simulink model "Robot_Falling_Down_Stairs"  (R2024b)
settings: Solver=ode23t  StartTime=0.0  StopTime=4  RelTol=1e-3  AbsTol=1e-2  EnableMultiTasking=on
undefined symbols (not defined in the file): points, X, Y, Z
libraries: sm_lib x981, PC_GS_SSMB x30, nesl_utility x4
(part 4 of 22)

== system Robot_Falling_Down_Stairs/Sagte_Woef/FLLeg/Joint ==
  Revolute Joint1 : sm_lib/Joints/Revolute Joint  JointMode=Normal  CompositeWrenchDir=FollowerOnBase  CompositeWrenchFrame=BaseFrame  SenseConstraintForce=off  SenseConstraintTorque=off  SenseTotalForce=off  SenseTotalTorque=off  PositionTargetSpecify=on  PositionTargetPriority=High  PositionTargetValue=-50  PositionTargetValueUnits=deg  VelocityTargetSpecify=off  VelocityTargetPriority=High  VelocityTargetValue=0  VelocityTargetValueUnits=deg/s  EquilibriumPosition=0  EquilibriumPositionUnits=deg  SpringStiffness=0  SpringStiffnessUnits=N*m/deg  DampingCoefficient=1  DampingCoefficientUnits=N*m/(deg/s)  LowerLimitSpecify=off  LowerLimitBound=-90  LowerLimitBoundUnits=deg  LowerLimitStiffness=1e4  LowerLimitStiffnessUnits=N*m/deg  LowerLimitDamping=10  LowerLimitDampingUnits=N*m/(deg/s)  LowerLimitTransitionRegionWidth=0.1  LowerLimitTransitionRegionWidthUnits=deg  UpperLimitSpecify=off  UpperLimitBound=90  UpperLimitBoundUnits=deg  UpperLimitStiffness=1e4  UpperLimitStiffnessUnits=N*m/deg  UpperLimitDamping=10  UpperLimitDampingUnits=N*m/(deg/s)  UpperLimitTransitionRegionWidth=0.1  UpperLimitTransitionRegionWidthUnits=deg  TorqueActuationMode=NoTorque  MotionActuationMode=ComputedMotion  SensePosition=off  SenseVelocity=off  SenseAcceleration=off  SenseTorqueForce=off  SenseLowerLimitTorqueForce=off  SenseUpperLimitTorqueForce=off
  Rigid Transform2 : sm_lib/Frames and Transforms/Rigid Transform  RotationMethod=StandardAxis  FollAlignAxisA=+X  BaseAlignAxisA=+Y  FollAlignAxisB=+Y  BaseAlignAxisB=+Z  RotationStandardAxis=+X  RotationAngle=90  RotationAngleUnits=deg  RotationArbitraryAxis=[0 0 1]  RotationSequenceAxes=FollowerAxes  RotationSequence=XYX  RotationSequenceAngles=[0 0 0]  RotationSequenceAnglesUnits=deg  RotationMatrix=[1 0 0;0 1 0;0 0 1]  Quaternion=[1 0 0 0]  TranslationMethod=None  TranslationCartesianOffset=[0 0 0]  TranslationCartesianOffsetUnits=m  TranslationStandardAxis=+Z  TranslationStandardOffset=0  TranslationStandardOffsetUnits=m  TranslationROffset=0  TranslationROffsetUnits=m  TranslationThetaOffset=0  TranslationThetaOffsetUnits=deg  TranslationZOffset=0  TranslationZOffsetUnits=m
  Rigid Transform3 : sm_lib/Frames and Transforms/Rigid Transform  RotationMethod=StandardAxis  FollAlignAxisA=+X  BaseAlignAxisA=+Y  FollAlignAxisB=+Y  BaseAlignAxisB=+Z  RotationStandardAxis=+X  RotationAngle=90  RotationAngleUnits=deg  RotationArbitraryAxis=[0 0 1]  RotationSequenceAxes=FollowerAxes  RotationSequence=XYX  RotationSequenceAngles=[0 0 0]  RotationSequenceAnglesUnits=deg  RotationMatrix=[1 0 0;0 1 0;0 0 1]  Quaternion=[1 0 0 0]  TranslationMethod=None  TranslationCartesianOffset=[0 0 0]  TranslationCartesianOffsetUnits=m  TranslationStandardAxis=+Z  TranslationStandardOffset=0  TranslationStandardOffsetUnits=m  TranslationROffset=0  TranslationROffsetUnits=m  TranslationThetaOffset=0  TranslationThetaOffsetUnits=deg  TranslationZOffset=0  TranslationZOffsetUnits=m
  Conn1 : PMIOPort  Side=Right
  Top : PMIOPort  Port=2  Side=Left
  connections:
  Rigid Transform3/RConn1 -- Top/RConn1
  Rigid Transform2/LConn1 -- Revolute Joint1/LConn1
  Revolute Joint1/RConn1 -- Rigid Transform3/LConn1
  Conn1/RConn1 -- Rigid Transform2/RConn1

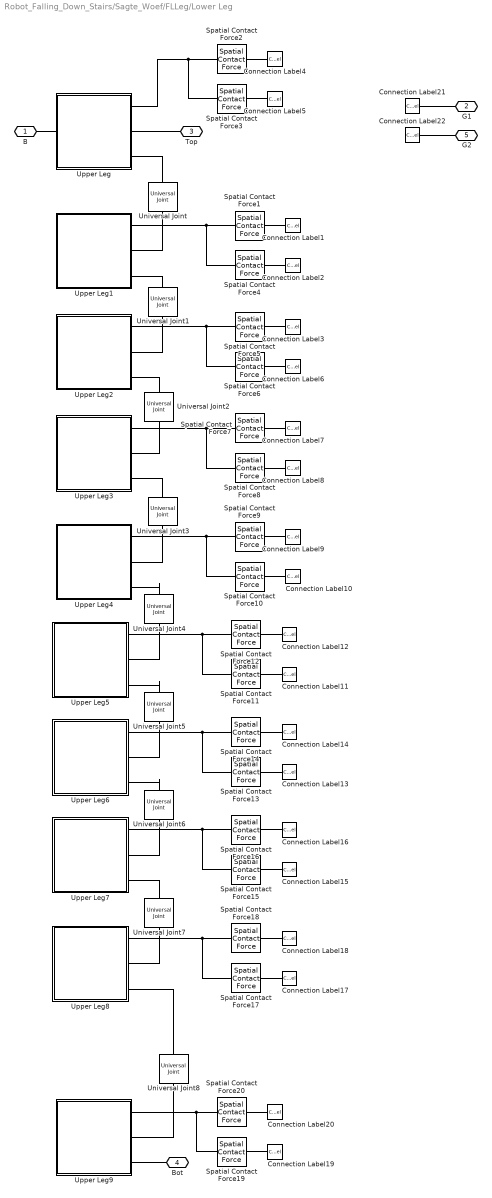
== system Robot_Falling_Down_Stairs/Sagte_Woef/FLLeg/Lower Leg ==
  Connection Label{1,3..4,7,9,12,14,16,18,20..21} (11 alike) : ConnectionLabel  Label=G1
  Connection Label{2,5..6,8,10..11,13,15,17,19,22} (11 alike) : ConnectionLabel  Label=G2
  Spatial Contact Force{1..20} (20 alike) : sm_lib/Forces and Torques/Spatial Contact Force  NormalForceType=SmoothSpringDamper  NormalStiffness=1e6  NormalStiffnessUnits=N/m  NormalDamping=1e3  NormalDampingUnits=N/(m/s)  NormalTransitionRegionWidth=1e-2  NormalTransitionRegionWidthUnits=m  FrictionType=SmoothStickSlip  CoefficientOfStaticFriction=0.5  CoefficientOfDynamicFriction=0.3  FrictionalCriticalVelocity=1e-3  FrictionalCriticalVelocityUnits=m/s  SenseContactSignal=off  SensePenetrationDepth=off  SenseSeparationDistance=off  SenseNormalForceMagnitude=off  SenseFrictionalForceMagnitude=off  SenseNormalVelocity=off  SenseTangentialVelocity=off  SenseContactBaseRotation=off  SenseContactBaseTranslation=off  SenseContactFollowerRotation=off  SenseContactFollowerTranslation=off  ZeroCrossDetectContactStartAndEnd=off
  Universal Joint{,1..8} (9 alike) : sm_lib/Joints/Universal Joint  JointMode=Normal  CompositeWrenchDir=FollowerOnBase  CompositeWrenchFrame=BaseFrame  SenseConstraintForce=off  SenseConstraintTorque=off  SenseTotalForce=off  SenseTotalTorque=off  RxPositionTargetSpecify=off  RxPositionTargetPriority=High  RxPositionTargetValue=0  RxPositionTargetValueUnits=deg  RxVelocityTargetSpecify=off  RxVelocityTargetPriority=High  RxVelocityTargetValue=0  RxVelocityTargetValueUnits=deg/s  RxEquilibriumPosition=0  RxEquilibriumPositionUnits=deg  RxSpringStiffness=100  RxSpringStiffnessUnits=N*m/deg  RxDampingCoefficient=.1  RxDampingCoefficientUnits=N*m/(deg/s)  RxLowerLimitSpecify=off  RxLowerLimitBound=-90  RxLowerLimitBoundUnits=deg  RxLowerLimitStiffness=1e4  RxLowerLimitStiffnessUnits=N*m/deg  RxLowerLimitDamping=10  RxLowerLimitDampingUnits=N*m/(deg/s)  RxLowerLimitTransitionRegionWidth=0.1  RxLowerLimitTransitionRegionWidthUnits=deg  RxUpperLimitSpecify=off  RxUpperLimitBound=90  RxUpperLimitBoundUnits=deg  RxUpperLimitStiffness=1e4  RxUpperLimitStiffnessUnits=N*m/deg  RxUpperLimitDamping=10  RxUpperLimitDampingUnits=N*m/(deg/s)  RxUpperLimitTransitionRegionWidth=0.1  RxUpperLimitTransitionRegionWidthUnits=deg  RxTorqueActuationMode=NoTorque  RxMotionActuationMode=ComputedMotion  RxSensePosition=off  RxSenseVelocity=off  RxSenseAcceleration=off  RxSenseTorqueForce=off  RxSenseLowerLimitTorqueForce=off  RxSenseUpperLimitTorqueForce=off  RyPositionTargetSpecify=off  RyPositionTargetPriority=High  RyPositionTargetValue=0  RyPositionTargetValueUnits=deg  RyVelocityTargetSpecify=off  RyVelocityTargetPriority=High  RyVelocityTargetValue=0  RyVelocityTargetValueUnits=deg/s  RyEquilibriumPosition=0  RyEquilibriumPositionUnits=deg  RySpringStiffness=100  RySpringStiffnessUnits=N*m/deg  RyDampingCoefficient=.1  RyDampingCoefficientUnits=N*m/(deg/s)  RyLowerLimitSpecify=off  RyLowerLimitBound=-90  RyLowerLimitBoundUnits=deg  RyLowerLimitStiffness=1e4  RyLowerLimitStiffnessUnits=N*m/deg  RyLowerLimitDamping=10  RyLowerLimitDampingUnits=N*m/(deg/s)  RyLowerLimitTransitionRegionWidth=0.1  RyLowerLimitTransitionRegionWidthUnits=deg  RyUpperLimitSpecify=off  RyUpperLimitBound=90  RyUpperLimitBoundUnits=deg  RyUpperLimitStiffness=1e4  RyUpperLimitStiffnessUnits=N*m/deg  RyUpperLimitDamping=10  RyUpperLimitDampingUnits=N*m/(deg/s)  RyUpperLimitTransitionRegionWidth=0.1  RyUpperLimitTransitionRegionWidthUnits=deg  RyTorqueActuationMode=NoTorque  RyMotionActuationMode=ComputedMotion  RySensePosition=off  RySenseVelocity=off  RySenseAcceleration=off  RySenseTorqueForce=off  RySenseLowerLimitTorqueForce=off  RySenseUpperLimitTorqueForce=off
  Upper Leg : Subsystem  AncestorBlock=PC_GS_SSMB/PC_Cylinder_F  mask{radius=30, height=40, numPoints=9*3*3, numCircumferencePoints=0, numFacePoints=20, patchColor=[0.1940, 0.4840, 0.5560], markerColor=[0 0.4470 0.7410]}
      mask prompts: radius "Radius of the cylider [mm]"; height "Height of the cylinder [mm]"; numPoints "Total number of points for the curved surface using the Fibonacci lattice"; numCircumferencePoints "Number of points on the circumference of each disc"; numFacePoints "Number of points on each disc surface using the Fibonacci lattice"; patchColor "RGB color for the patches"; markerColor "RGB color for the markers"
      mask init:
      | [points, imageMatrix] = generateCylinderPointCloudFibonacci(radius, height, numPoints, numCircumferencePoints, numFacePoints, patchColor, markerColor);
      mask Display: image(imageMatrix);
  Upper Leg1 : Subsystem  AncestorBlock=PC_GS_SSMB/PC_Cylinder_F  mask{radius=30, height=40, numPoints=9*3*3, numCircumferencePoints=0, numFacePoints=0, patchColor=[0.1940, 0.4840, 0.5560], markerColor=[0 0.4470 0.7410]}
      mask prompts: radius "Radius of the cylider [mm]"; height "Height of the cylinder [mm]"; numPoints "Total number of points for the curved surface using the Fibonacci lattice"; numCircumferencePoints "Number of points on the circumference of each disc"; numFacePoints "Number of points on each disc surface using the Fibonacci lattice"; patchColor "RGB color for the patches"; markerColor "RGB color for the markers"
      mask init:
      | [points, imageMatrix] = generateCylinderPointCloudFibonacci(radius, height, numPoints, numCircumferencePoints, numFacePoints, patchColor, markerColor);
      mask Display: image(imageMatrix);
  Upper Leg2 : Subsystem  AncestorBlock=PC_GS_SSMB/PC_Cylinder_F  mask{radius=30, height=40, numPoints=9*3*3, numCircumferencePoints=0, numFacePoints=0, patchColor=[0.1940, 0.4840, 0.5560], markerColor=[0 0.4470 0.7410]}
      mask prompts: radius "Radius of the cylider [mm]"; height "Height of the cylinder [mm]"; numPoints "Total number of points for the curved surface using the Fibonacci lattice"; numCircumferencePoints "Number of points on the circumference of each disc"; numFacePoints "Number of points on each disc surface using the Fibonacci lattice"; patchColor "RGB color for the patches"; markerColor "RGB color for the markers"
      mask init:
      | [points, imageMatrix] = generateCylinderPointCloudFibonacci(radius, height, numPoints, numCircumferencePoints, numFacePoints, patchColor, markerColor);
      mask Display: image(imageMatrix);
  Upper Leg3 : Subsystem  AncestorBlock=PC_GS_SSMB/PC_Cylinder_F  mask{radius=30, height=40, numPoints=9*3*3, numCircumferencePoints=0, numFacePoints=0, patchColor=[0.1940, 0.4840, 0.5560], markerColor=[0 0.4470 0.7410]}
      mask prompts: radius "Radius of the cylider [mm]"; height "Height of the cylinder [mm]"; numPoints "Total number of points for the curved surface using the Fibonacci lattice"; numCircumferencePoints "Number of points on the circumference of each disc"; numFacePoints "Number of points on each disc surface using the Fibonacci lattice"; patchColor "RGB color for the patches"; markerColor "RGB color for the markers"
      mask init:
      | [points, imageMatrix] = generateCylinderPointCloudFibonacci(radius, height, numPoints, numCircumferencePoints, numFacePoints, patchColor, markerColor);
      mask Display: image(imageMatrix);
  Upper Leg4 : Subsystem  AncestorBlock=PC_GS_SSMB/PC_Cylinder_F  mask{radius=30, height=40, numPoints=9*3*3, numCircumferencePoints=0, numFacePoints=0, patchColor=[0.1940, 0.4840, 0.5560], markerColor=[0 0.4470 0.7410]}
      mask prompts: radius "Radius of the cylider [mm]"; height "Height of the cylinder [mm]"; numPoints "Total number of points for the curved surface using the Fibonacci lattice"; numCircumferencePoints "Number of points on the circumference of each disc"; numFacePoints "Number of points on each disc surface using the Fibonacci lattice"; patchColor "RGB color for the patches"; markerColor "RGB color for the markers"
      mask init:
      | [points, imageMatrix] = generateCylinderPointCloudFibonacci(radius, height, numPoints, numCircumferencePoints, numFacePoints, patchColor, markerColor);
      mask Display: image(imageMatrix);
  Upper Leg5 : Subsystem  AncestorBlock=PC_GS_SSMB/PC_Cylinder_F  mask{radius=30, height=40, numPoints=9*3*3, numCircumferencePoints=0, numFacePoints=0, patchColor=[0.1940, 0.4840, 0.5560], markerColor=[0 0.4470 0.7410]}
      mask prompts: radius "Radius of the cylider [mm]"; height "Height of the cylinder [mm]"; numPoints "Total number of points for the curved surface using the Fibonacci lattice"; numCircumferencePoints "Number of points on the circumference of each disc"; numFacePoints "Number of points on each disc surface using the Fibonacci lattice"; patchColor "RGB color for the patches"; markerColor "RGB color for the markers"
      mask init:
      | [points, imageMatrix] = generateCylinderPointCloudFibonacci(radius, height, numPoints, numCircumferencePoints, numFacePoints, patchColor, markerColor);
      mask Display: image(imageMatrix);
  Upper Leg6 : Subsystem  AncestorBlock=PC_GS_SSMB/PC_Cylinder_F  mask{radius=30, height=40, numPoints=9*3*3, numCircumferencePoints=0, numFacePoints=0, patchColor=[0.1940, 0.4840, 0.5560], markerColor=[0 0.4470 0.7410]}
      mask prompts: radius "Radius of the cylider [mm]"; height "Height of the cylinder [mm]"; numPoints "Total number of points for the curved surface using the Fibonacci lattice"; numCircumferencePoints "Number of points on the circumference of each disc"; numFacePoints "Number of points on each disc surface using the Fibonacci lattice"; patchColor "RGB color for the patches"; markerColor "RGB color for the markers"
      mask init:
      | [points, imageMatrix] = generateCylinderPointCloudFibonacci(radius, height, numPoints, numCircumferencePoints, numFacePoints, patchColor, markerColor);
      mask Display: image(imageMatrix);
  Upper Leg7 : Subsystem  AncestorBlock=PC_GS_SSMB/PC_Cylinder_F  mask{radius=30, height=40, numPoints=9*3*3, numCircumferencePoints=0, numFacePoints=0, patchColor=[0.1940, 0.4840, 0.5560], markerColor=[0 0.4470 0.7410]}
      mask prompts: radius "Radius of the cylider [mm]"; height "Height of the cylinder [mm]"; numPoints "Total number of points for the curved surface using the Fibonacci lattice"; numCircumferencePoints "Number of points on the circumference of each disc"; numFacePoints "Number of points on each disc surface using the Fibonacci lattice"; patchColor "RGB color for the patches"; markerColor "RGB color for the markers"
      mask init:
      | [points, imageMatrix] = generateCylinderPointCloudFibonacci(radius, height, numPoints, numCircumferencePoints, numFacePoints, patchColor, markerColor);
      mask Display: image(imageMatrix);
  Upper Leg8 : Subsystem  AncestorBlock=PC_GS_SSMB/PC_Cylinder_F  mask{radius=30, height=40, numPoints=9*3*3, numCircumferencePoints=0, numFacePoints=0, patchColor=[0.1940, 0.4840, 0.5560], markerColor=[0 0.4470 0.7410]}
      mask prompts: radius "Radius of the cylider [mm]"; height "Height of the cylinder [mm]"; numPoints "Total number of points for the curved surface using the Fibonacci lattice"; numCircumferencePoints "Number of points on the circumference of each disc"; numFacePoints "Number of points on each disc surface using the Fibonacci lattice"; patchColor "RGB color for the patches"; markerColor "RGB color for the markers"
      mask init:
      | [points, imageMatrix] = generateCylinderPointCloudFibonacci(radius, height, numPoints, numCircumferencePoints, numFacePoints, patchColor, markerColor);
      mask Display: image(imageMatrix);
  Upper Leg9 : Subsystem  AncestorBlock=PC_GS_SSMB/PC_Cylinder_F  mask{radius=30, height=40, numPoints=9*3*3, numCircumferencePoints=0, numFacePoints=20, patchColor=[0.1940, 0.4840, 0.5560], markerColor=[0 0.4470 0.7410]}
      mask prompts: radius "Radius of the cylider [mm]"; height "Height of the cylinder [mm]"; numPoints "Total number of points for the curved surface using the Fibonacci lattice"; numCircumferencePoints "Number of points on the circumference of each disc"; numFacePoints "Number of points on each disc surface using the Fibonacci lattice"; patchColor "RGB color for the patches"; markerColor "RGB color for the markers"
      mask init:
      | [points, imageMatrix] = generateCylinderPointCloudFibonacci(radius, height, numPoints, numCircumferencePoints, numFacePoints, patchColor, markerColor);
      mask Display: image(imageMatrix);
  B : PMIOPort  Side=Left
  G1 : PMIOPort  Port=2  Side=Right
  Top : PMIOPort  Port=3  Side=Right
  Bot : PMIOPort  Port=4  Side=Right
  G2 : PMIOPort  Port=5  Side=Right
  connections:
  Connection Label22/LConn1 -- G2/RConn1
  Connection Label21/LConn1 -- G1/RConn1
  Universal Joint1/LConn1 -- Upper Leg1/RConn3
  Universal Joint2/LConn1 -- Upper Leg2/RConn3
  Universal Joint3/LConn1 -- Upper Leg3/RConn3
  Universal Joint4/LConn1 -- Upper Leg4/RConn3
  Universal Joint5/LConn1 -- Upper Leg5/RConn3
  Universal Joint6/LConn1 -- Upper Leg6/RConn3
  Universal Joint7/LConn1 -- Upper Leg7/RConn3
  Universal Joint8/LConn1 -- Upper Leg8/RConn3
  Spatial Contact Force19/LConn1 -- Spatial Contact Force20/LConn1 -- Upper Leg9/RConn1
  Connection Label19/LConn1 -- Spatial Contact Force19/RConn1
  Spatial Contact Force20/RConn1 -- Connection Label20/LConn1
  Upper Leg9/RConn2 -- Universal Joint8/RConn1
  Spatial Contact Force18/RConn1 -- Connection Label18/LConn1
  Connection Label17/LConn1 -- Spatial Contact Force17/RConn1
  Universal Joint7/RConn1 -- Upper Leg8/RConn2
  Spatial Contact Force17/LConn1 -- Upper Leg8/RConn1 -- Spatial Contact Force18/LConn1
  Universal Joint6/RConn1 -- Upper Leg7/RConn2
  Spatial Contact Force16/RConn1 -- Connection Label16/LConn1
  Connection Label15/LConn1 -- Spatial Contact Force15/RConn1
  Upper Leg7/RConn1 -- Spatial Contact Force15/LConn1 -- Spatial Contact Force16/LConn1
  Connection Label13/LConn1 -- Spatial Contact Force13/RConn1
  Spatial Contact Force14/RConn1 -- Connection Label14/LConn1
  Universal Joint5/RConn1 -- Upper Leg6/RConn2
  Spatial Contact Force13/LConn1 -- Upper Leg6/RConn1 -- Spatial Contact Force14/LConn1
  Universal Joint4/RConn1 -- Upper Leg5/RConn2
  Connection Label11/LConn1 -- Spatial Contact Force11/RConn1
  Spatial Contact Force12/RConn1 -- Connection Label12/LConn1
  Upper Leg5/RConn1 -- Spatial Contact Force11/LConn1 -- Spatial Contact Force12/LConn1
  Spatial Contact Force9/RConn1 -- Connection Label9/LConn1
  Universal Joint3/RConn1 -- Upper Leg4/RConn2
  Connection Label10/LConn1 -- Spatial Contact Force10/RConn1
  Spatial Contact Force10/LConn1 -- Upper Leg4/RConn1 -- Spatial Contact Force9/LConn1
  Universal Joint2/RConn1 -- Upper Leg3/RConn2
  Connection Label8/LConn1 -- Spatial Contact Force8/RConn1
  Spatial Contact Force7/RConn1 -- Connection Label7/LConn1
  Upper Leg3/RConn1 -- Spatial Contact Force8/LConn1 -- Spatial Contact Force7/LConn1
  Universal Joint1/RConn1 -- Upper Leg2/RConn2
  Spatial Contact Force5/RConn1 -- Connection Label3/LConn1
  Connection Label6/LConn1 -- Spatial Contact Force6/RConn1
  Spatial Contact Force6/LConn1 -- Upper Leg2/RConn1 -- Spatial Contact Force5/LConn1
  Connection Label2/LConn1 -- Spatial Contact Force4/RConn1
  Spatial Contact Force1/RConn1 -- Connection Label1/LConn1
  Upper Leg1/RConn1 -- Spatial Contact Force4/LConn1 -- Spatial Contact Force1/LConn1
  Universal Joint/RConn1 -- Upper Leg1/RConn2
  Upper Leg/RConn3 -- Universal Joint/LConn1
  Connection Label5/LConn1 -- Spatial Contact Force3/RConn1
  Spatial Contact Force2/RConn1 -- Connection Label4/LConn1
  Upper Leg/RConn1 -- Spatial Contact Force2/LConn1 -- Spatial Contact Force3/LConn1
  Bot/RConn1 -- Upper Leg9/RConn3
  Upper Leg/LConn1 -- B/RConn1
  Upper Leg/RConn2 -- Top/RConn1

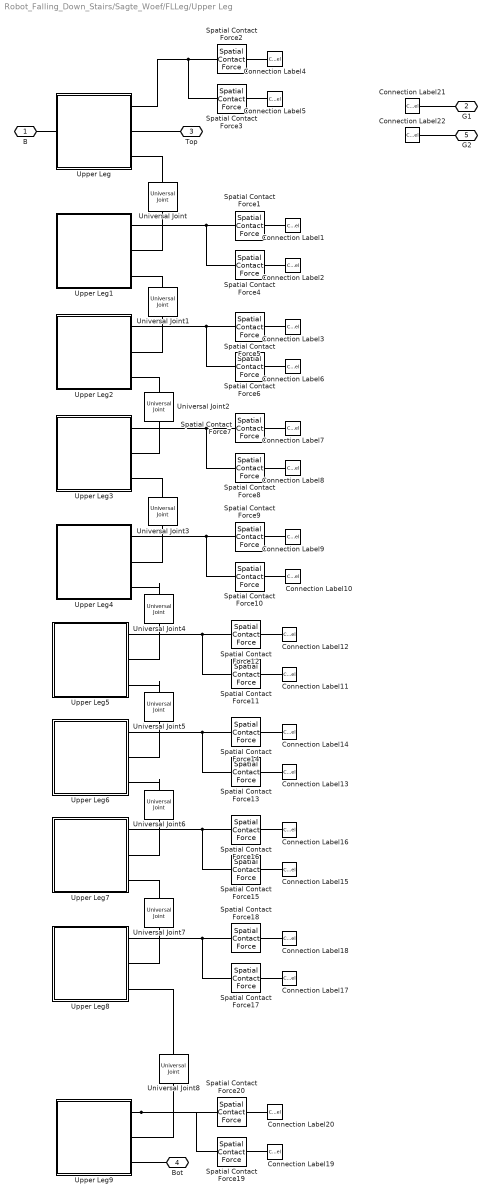
== system Robot_Falling_Down_Stairs/Sagte_Woef/FLLeg/Upper Leg ==
  Connection Label{1,3..4,7,9,12,14,16,18,20..21} (11 alike) : ConnectionLabel  Label=G1
  Connection Label{2,5..6,8,10..11,13,15,17,19,22} (11 alike) : ConnectionLabel  Label=G2
  Spatial Contact Force{1..20} (20 alike) : sm_lib/Forces and Torques/Spatial Contact Force  NormalForceType=SmoothSpringDamper  NormalStiffness=1e6  NormalStiffnessUnits=N/m  NormalDamping=1e3  NormalDampingUnits=N/(m/s)  NormalTransitionRegionWidth=1e-2  NormalTransitionRegionWidthUnits=m  FrictionType=SmoothStickSlip  CoefficientOfStaticFriction=0.5  CoefficientOfDynamicFriction=0.3  FrictionalCriticalVelocity=1e-3  FrictionalCriticalVelocityUnits=m/s  SenseContactSignal=off  SensePenetrationDepth=off  SenseSeparationDistance=off  SenseNormalForceMagnitude=off  SenseFrictionalForceMagnitude=off  SenseNormalVelocity=off  SenseTangentialVelocity=off  SenseContactBaseRotation=off  SenseContactBaseTranslation=off  SenseContactFollowerRotation=off  SenseContactFollowerTranslation=off  ZeroCrossDetectContactStartAndEnd=off
  Universal Joint{,1..8} (9 alike) : sm_lib/Joints/Universal Joint  JointMode=Normal  CompositeWrenchDir=FollowerOnBase  CompositeWrenchFrame=BaseFrame  SenseConstraintForce=off  SenseConstraintTorque=off  SenseTotalForce=off  SenseTotalTorque=off  RxPositionTargetSpecify=off  RxPositionTargetPriority=High  RxPositionTargetValue=0  RxPositionTargetValueUnits=deg  RxVelocityTargetSpecify=off  RxVelocityTargetPriority=High  RxVelocityTargetValue=0  RxVelocityTargetValueUnits=deg/s  RxEquilibriumPosition=0  RxEquilibriumPositionUnits=deg  RxSpringStiffness=100  RxSpringStiffnessUnits=N*m/deg  RxDampingCoefficient=.1  RxDampingCoefficientUnits=N*m/(deg/s)  RxLowerLimitSpecify=off  RxLowerLimitBound=-90  RxLowerLimitBoundUnits=deg  RxLowerLimitStiffness=1e4  RxLowerLimitStiffnessUnits=N*m/deg  RxLowerLimitDamping=10  RxLowerLimitDampingUnits=N*m/(deg/s)  RxLowerLimitTransitionRegionWidth=0.1  RxLowerLimitTransitionRegionWidthUnits=deg  RxUpperLimitSpecify=off  RxUpperLimitBound=90  RxUpperLimitBoundUnits=deg  RxUpperLimitStiffness=1e4  RxUpperLimitStiffnessUnits=N*m/deg  RxUpperLimitDamping=10  RxUpperLimitDampingUnits=N*m/(deg/s)  RxUpperLimitTransitionRegionWidth=0.1  RxUpperLimitTransitionRegionWidthUnits=deg  RxTorqueActuationMode=NoTorque  RxMotionActuationMode=ComputedMotion  RxSensePosition=off  RxSenseVelocity=off  RxSenseAcceleration=off  RxSenseTorqueForce=off  RxSenseLowerLimitTorqueForce=off  RxSenseUpperLimitTorqueForce=off  RyPositionTargetSpecify=off  RyPositionTargetPriority=High  RyPositionTargetValue=0  RyPositionTargetValueUnits=deg  RyVelocityTargetSpecify=off  RyVelocityTargetPriority=High  RyVelocityTargetValue=0  RyVelocityTargetValueUnits=deg/s  RyEquilibriumPosition=0  RyEquilibriumPositionUnits=deg  RySpringStiffness=100  RySpringStiffnessUnits=N*m/deg  RyDampingCoefficient=.1  RyDampingCoefficientUnits=N*m/(deg/s)  RyLowerLimitSpecify=off  RyLowerLimitBound=-90  RyLowerLimitBoundUnits=deg  RyLowerLimitStiffness=1e4  RyLowerLimitStiffnessUnits=N*m/deg  RyLowerLimitDamping=10  RyLowerLimitDampingUnits=N*m/(deg/s)  RyLowerLimitTransitionRegionWidth=0.1  RyLowerLimitTransitionRegionWidthUnits=deg  RyUpperLimitSpecify=off  RyUpperLimitBound=90  RyUpperLimitBoundUnits=deg  RyUpperLimitStiffness=1e4  RyUpperLimitStiffnessUnits=N*m/deg  RyUpperLimitDamping=10  RyUpperLimitDampingUnits=N*m/(deg/s)  RyUpperLimitTransitionRegionWidth=0.1  RyUpperLimitTransitionRegionWidthUnits=deg  RyTorqueActuationMode=NoTorque  RyMotionActuationMode=ComputedMotion  RySensePosition=off  RySenseVelocity=off  RySenseAcceleration=off  RySenseTorqueForce=off  RySenseLowerLimitTorqueForce=off  RySenseUpperLimitTorqueForce=off
  Upper Leg : Subsystem  AncestorBlock=PC_GS_SSMB/PC_Cylinder_F  mask{radius=40, height=30, numPoints=9*3*3, numCircumferencePoints=0, numFacePoints=20, patchColor=[0.4940, 0.1840, 0.5560], markerColor=[0 0.4470 0.7410]}
      mask prompts: radius "Radius of the cylider [mm]"; height "Height of the cylinder [mm]"; numPoints "Total number of points for the curved surface using the Fibonacci lattice"; numCircumferencePoints "Number of points on the circumference of each disc"; numFacePoints "Number of points on each disc surface using the Fibonacci lattice"; patchColor "RGB color for the patches"; markerColor "RGB color for the markers"
      mask init:
      | [points, imageMatrix] = generateCylinderPointCloudFibonacci(radius, height, numPoints, numCircumferencePoints, numFacePoints, patchColor, markerColor);
      mask Display: image(imageMatrix);
  Upper Leg1 : Subsystem  AncestorBlock=PC_GS_SSMB/PC_Cylinder_F  mask{radius=40, height=30, numPoints=9*3*3, numCircumferencePoints=0, numFacePoints=0, patchColor=[0.4940, 0.1840, 0.5560], markerColor=[0 0.4470 0.7410]}
      mask prompts: radius "Radius of the cylider [mm]"; height "Height of the cylinder [mm]"; numPoints "Total number of points for the curved surface using the Fibonacci lattice"; numCircumferencePoints "Number of points on the circumference of each disc"; numFacePoints "Number of points on each disc surface using the Fibonacci lattice"; patchColor "RGB color for the patches"; markerColor "RGB color for the markers"
      mask init:
      | [points, imageMatrix] = generateCylinderPointCloudFibonacci(radius, height, numPoints, numCircumferencePoints, numFacePoints, patchColor, markerColor);
      mask Display: image(imageMatrix);
  Upper Leg2 : Subsystem  AncestorBlock=PC_GS_SSMB/PC_Cylinder_F  mask{radius=40, height=30, numPoints=9*3*3, numCircumferencePoints=0, numFacePoints=0, patchColor=[0.4940, 0.1840, 0.5560], markerColor=[0 0.4470 0.7410]}
      mask prompts: radius "Radius of the cylider [mm]"; height "Height of the cylinder [mm]"; numPoints "Total number of points for the curved surface using the Fibonacci lattice"; numCircumferencePoints "Number of points on the circumference of each disc"; numFacePoints "Number of points on each disc surface using the Fibonacci lattice"; patchColor "RGB color for the patches"; markerColor "RGB color for the markers"
      mask init:
      | [points, imageMatrix] = generateCylinderPointCloudFibonacci(radius, height, numPoints, numCircumferencePoints, numFacePoints, patchColor, markerColor);
      mask Display: image(imageMatrix);
  Upper Leg3 : Subsystem  AncestorBlock=PC_GS_SSMB/PC_Cylinder_F  mask{radius=40, height=30, numPoints=9*3*3, numCircumferencePoints=0, numFacePoints=0, patchColor=[0.4940, 0.1840, 0.5560], markerColor=[0 0.4470 0.7410]}
      mask prompts: radius "Radius of the cylider [mm]"; height "Height of the cylinder [mm]"; numPoints "Total number of points for the curved surface using the Fibonacci lattice"; numCircumferencePoints "Number of points on the circumference of each disc"; numFacePoints "Number of points on each disc surface using the Fibonacci lattice"; patchColor "RGB color for the patches"; markerColor "RGB color for the markers"
      mask init:
      | [points, imageMatrix] = generateCylinderPointCloudFibonacci(radius, height, numPoints, numCircumferencePoints, numFacePoints, patchColor, markerColor);
      mask Display: image(imageMatrix);
  Upper Leg4 : Subsystem  AncestorBlock=PC_GS_SSMB/PC_Cylinder_F  mask{radius=40, height=30, numPoints=9*3*3, numCircumferencePoints=0, numFacePoints=0, patchColor=[0.4940, 0.1840, 0.5560], markerColor=[0 0.4470 0.7410]}
      mask prompts: radius "Radius of the cylider [mm]"; height "Height of the cylinder [mm]"; numPoints "Total number of points for the curved surface using the Fibonacci lattice"; numCircumferencePoints "Number of points on the circumference of each disc"; numFacePoints "Number of points on each disc surface using the Fibonacci lattice"; patchColor "RGB color for the patches"; markerColor "RGB color for the markers"
      mask init:
      | [points, imageMatrix] = generateCylinderPointCloudFibonacci(radius, height, numPoints, numCircumferencePoints, numFacePoints, patchColor, markerColor);
      mask Display: image(imageMatrix);
  Upper Leg5 : Subsystem  AncestorBlock=PC_GS_SSMB/PC_Cylinder_F  mask{radius=40, height=30, numPoints=9*3*3, numCircumferencePoints=0, numFacePoints=0, patchColor=[0.4940, 0.1840, 0.5560], markerColor=[0 0.4470 0.7410]}
      mask prompts: radius "Radius of the cylider [mm]"; height "Height of the cylinder [mm]"; numPoints "Total number of points for the curved surface using the Fibonacci lattice"; numCircumferencePoints "Number of points on the circumference of each disc"; numFacePoints "Number of points on each disc surface using the Fibonacci lattice"; patchColor "RGB color for the patches"; markerColor "RGB color for the markers"
      mask init:
      | [points, imageMatrix] = generateCylinderPointCloudFibonacci(radius, height, numPoints, numCircumferencePoints, numFacePoints, patchColor, markerColor);
      mask Display: image(imageMatrix);
  Upper Leg6 : Subsystem  AncestorBlock=PC_GS_SSMB/PC_Cylinder_F  mask{radius=40, height=30, numPoints=9*3*3, numCircumferencePoints=0, numFacePoints=0, patchColor=[0.4940, 0.1840, 0.5560], markerColor=[0 0.4470 0.7410]}
      mask prompts: radius "Radius of the cylider [mm]"; height "Height of the cylinder [mm]"; numPoints "Total number of points for the curved surface using the Fibonacci lattice"; numCircumferencePoints "Number of points on the circumference of each disc"; numFacePoints "Number of points on each disc surface using the Fibonacci lattice"; patchColor "RGB color for the patches"; markerColor "RGB color for the markers"
      mask init:
      | [points, imageMatrix] = generateCylinderPointCloudFibonacci(radius, height, numPoints, numCircumferencePoints, numFacePoints, patchColor, markerColor);
      mask Display: image(imageMatrix);
  Upper Leg7 : Subsystem  AncestorBlock=PC_GS_SSMB/PC_Cylinder_F  mask{radius=40, height=30, numPoints=9*3*3, numCircumferencePoints=0, numFacePoints=0, patchColor=[0.4940, 0.1840, 0.5560], markerColor=[0 0.4470 0.7410]}
      mask prompts: radius "Radius of the cylider [mm]"; height "Height of the cylinder [mm]"; numPoints "Total number of points for the curved surface using the Fibonacci lattice"; numCircumferencePoints "Number of points on the circumference of each disc"; numFacePoints "Number of points on each disc surface using the Fibonacci lattice"; patchColor "RGB color for the patches"; markerColor "RGB color for the markers"
      mask init:
      | [points, imageMatrix] = generateCylinderPointCloudFibonacci(radius, height, numPoints, numCircumferencePoints, numFacePoints, patchColor, markerColor);
      mask Display: image(imageMatrix);
  Upper Leg8 : Subsystem  AncestorBlock=PC_GS_SSMB/PC_Cylinder_F  mask{radius=40, height=30, numPoints=9*3*3, numCircumferencePoints=0, numFacePoints=0, patchColor=[0.4940, 0.1840, 0.5560], markerColor=[0 0.4470 0.7410]}
      mask prompts: radius "Radius of the cylider [mm]"; height "Height of the cylinder [mm]"; numPoints "Total number of points for the curved surface using the Fibonacci lattice"; numCircumferencePoints "Number of points on the circumference of each disc"; numFacePoints "Number of points on each disc surface using the Fibonacci lattice"; patchColor "RGB color for the patches"; markerColor "RGB color for the markers"
      mask init:
      | [points, imageMatrix] = generateCylinderPointCloudFibonacci(radius, height, numPoints, numCircumferencePoints, numFacePoints, patchColor, markerColor);
      mask Display: image(imageMatrix);
  Upper Leg9 : Subsystem  AncestorBlock=PC_GS_SSMB/PC_Cylinder_F  mask{radius=40, height=30, numPoints=9*3*3, numCircumferencePoints=0, numFacePoints=20, patchColor=[0.4940, 0.1840, 0.5560], markerColor=[0 0.4470 0.7410]}
      mask prompts: radius "Radius of the cylider [mm]"; height "Height of the cylinder [mm]"; numPoints "Total number of points for the curved surface using the Fibonacci lattice"; numCircumferencePoints "Number of points on the circumference of each disc"; numFacePoints "Number of points on each disc surface using the Fibonacci lattice"; patchColor "RGB color for the patches"; markerColor "RGB color for the markers"
      mask init:
      | [points, imageMatrix] = generateCylinderPointCloudFibonacci(radius, height, numPoints, numCircumferencePoints, numFacePoints, patchColor, markerColor);
      mask Display: image(imageMatrix);
  B : PMIOPort  Side=Left
  G1 : PMIOPort  Port=2  Side=Right
  Top : PMIOPort  Port=3  Side=Right
  Bot : PMIOPort  Port=4  Side=Right
  G2 : PMIOPort  Port=5  Side=Right
  connections:
  Upper Leg/RConn2 -- Top/RConn1
  Upper Leg/LConn1 -- B/RConn1
  Bot/RConn1 -- Upper Leg9/RConn3
  Spatial Contact Force3/LConn1 -- Spatial Contact Force2/LConn1 -- Upper Leg/RConn1
  Spatial Contact Force2/RConn1 -- Connection Label4/LConn1
  Connection Label5/LConn1 -- Spatial Contact Force3/RConn1
  Upper Leg/RConn3 -- Universal Joint/LConn1
  Universal Joint/RConn1 -- Upper Leg1/RConn2
  Spatial Contact Force1/LConn1 -- Spatial Contact Force4/LConn1 -- Upper Leg1/RConn1
  Spatial Contact Force1/RConn1 -- Connection Label1/LConn1
  Connection Label2/LConn1 -- Spatial Contact Force4/RConn1
  Spatial Contact Force5/LConn1 -- Upper Leg2/RConn1 -- Spatial Contact Force6/LConn1
  Connection Label6/LConn1 -- Spatial Contact Force6/RConn1
  Spatial Contact Force5/RConn1 -- Connection Label3/LConn1
  Universal Joint1/RConn1 -- Upper Leg2/RConn2
  Spatial Contact Force7/LConn1 -- Spatial Contact Force8/LConn1 -- Upper Leg3/RConn1
  Spatial Contact Force7/RConn1 -- Connection Label7/LConn1
  Connection Label8/LConn1 -- Spatial Contact Force8/RConn1
  Universal Joint2/RConn1 -- Upper Leg3/RConn2
  Spatial Contact Force9/LConn1 -- Upper Leg4/RConn1 -- Spatial Contact Force10/LConn1
  Connection Label10/LConn1 -- Spatial Contact Force10/RConn1
  Universal Joint3/RConn1 -- Upper Leg4/RConn2
  Spatial Contact Force9/RConn1 -- Connection Label9/LConn1
  Spatial Contact Force12/LConn1 -- Spatial Contact Force11/LConn1 -- Upper Leg5/RConn1
  Spatial Contact Force12/RConn1 -- Connection Label12/LConn1
  Connection Label11/LConn1 -- Spatial Contact Force11/RConn1
  Universal Joint4/RConn1 -- Upper Leg5/RConn2
  Spatial Contact Force14/LConn1 -- Upper Leg6/RConn1 -- Spatial Contact Force13/LConn1
  Universal Joint5/RConn1 -- Upper Leg6/RConn2
  Spatial Contact Force14/RConn1 -- Connection Label14/LConn1
  Connection Label13/LConn1 -- Spatial Contact Force13/RConn1
  Spatial Contact Force16/LConn1 -- Spatial Contact Force15/LConn1 -- Upper Leg7/RConn1
  Connection Label15/LConn1 -- Spatial Contact Force15/RConn1
  Spatial Contact Force16/RConn1 -- Connection Label16/LConn1
  Universal Joint6/RConn1 -- Upper Leg7/RConn2
  Spatial Contact Force18/LConn1 -- Upper Leg8/RConn1 -- Spatial Contact Force17/LConn1
  Universal Joint7/RConn1 -- Upper Leg8/RConn2
  Connection Label17/LConn1 -- Spatial Contact Force17/RConn1
  Spatial Contact Force18/RConn1 -- Connection Label18/LConn1
  Upper Leg9/RConn2 -- Universal Joint8/RConn1
  Spatial Contact Force20/RConn1 -- Connection Label20/LConn1
  Connection Label19/LConn1 -- Spatial Contact Force19/RConn1
  Upper Leg9/RConn1 -- Spatial Contact Force20/LConn1 -- Spatial Contact Force19/LConn1
  Universal Joint8/LConn1 -- Upper Leg8/RConn3
  Universal Joint7/LConn1 -- Upper Leg7/RConn3
  Universal Joint6/LConn1 -- Upper Leg6/RConn3
  Universal Joint5/LConn1 -- Upper Leg5/RConn3
  Universal Joint4/LConn1 -- Upper Leg4/RConn3
  Universal Joint3/LConn1 -- Upper Leg3/RConn3
  Universal Joint2/LConn1 -- Upper Leg2/RConn3
  Universal Joint1/LConn1 -- Upper Leg1/RConn3
  Connection Label21/LConn1 -- G1/RConn1
  Connection Label22/LConn1 -- G2/RConn1

== system Robot_Falling_Down_Stairs/Sagte_Woef/FRLeg/Joint ==
  Revolute Joint1 : sm_lib/Joints/Revolute Joint  JointMode=Normal  CompositeWrenchDir=FollowerOnBase  CompositeWrenchFrame=BaseFrame  SenseConstraintForce=off  SenseConstraintTorque=off  SenseTotalForce=off  SenseTotalTorque=off  PositionTargetSpecify=on  PositionTargetPriority=High  PositionTargetValue=-50  PositionTargetValueUnits=deg  VelocityTargetSpecify=off  VelocityTargetPriority=High  VelocityTargetValue=0  VelocityTargetValueUnits=deg/s  EquilibriumPosition=0  EquilibriumPositionUnits=deg  SpringStiffness=0  SpringStiffnessUnits=N*m/deg  DampingCoefficient=1  DampingCoefficientUnits=N*m/(deg/s)  LowerLimitSpecify=off  LowerLimitBound=-90  LowerLimitBoundUnits=deg  LowerLimitStiffness=1e4  LowerLimitStiffnessUnits=N*m/deg  LowerLimitDamping=10  LowerLimitDampingUnits=N*m/(deg/s)  LowerLimitTransitionRegionWidth=0.1  LowerLimitTransitionRegionWidthUnits=deg  UpperLimitSpecify=off  UpperLimitBound=90  UpperLimitBoundUnits=deg  UpperLimitStiffness=1e4  UpperLimitStiffnessUnits=N*m/deg  UpperLimitDamping=10  UpperLimitDampingUnits=N*m/(deg/s)  UpperLimitTransitionRegionWidth=0.1  UpperLimitTransitionRegionWidthUnits=deg  TorqueActuationMode=NoTorque  MotionActuationMode=ComputedMotion  SensePosition=off  SenseVelocity=off  SenseAcceleration=off  SenseTorqueForce=off  SenseLowerLimitTorqueForce=off  SenseUpperLimitTorqueForce=off
  Rigid Transform2 : sm_lib/Frames and Transforms/Rigid Transform  RotationMethod=StandardAxis  FollAlignAxisA=+X  BaseAlignAxisA=+Y  FollAlignAxisB=+Y  BaseAlignAxisB=+Z  RotationStandardAxis=+X  RotationAngle=90  RotationAngleUnits=deg  RotationArbitraryAxis=[0 0 1]  RotationSequenceAxes=FollowerAxes  RotationSequence=XYX  RotationSequenceAngles=[0 0 0]  RotationSequenceAnglesUnits=deg  RotationMatrix=[1 0 0;0 1 0;0 0 1]  Quaternion=[1 0 0 0]  TranslationMethod=None  TranslationCartesianOffset=[0 0 0]  TranslationCartesianOffsetUnits=m  TranslationStandardAxis=+Z  TranslationStandardOffset=0  TranslationStandardOffsetUnits=m  TranslationROffset=0  TranslationROffsetUnits=m  TranslationThetaOffset=0  TranslationThetaOffsetUnits=deg  TranslationZOffset=0  TranslationZOffsetUnits=m
  Rigid Transform3 : sm_lib/Frames and Transforms/Rigid Transform  RotationMethod=StandardAxis  FollAlignAxisA=+X  BaseAlignAxisA=+Y  FollAlignAxisB=+Y  BaseAlignAxisB=+Z  RotationStandardAxis=+X  RotationAngle=90  RotationAngleUnits=deg  RotationArbitraryAxis=[0 0 1]  RotationSequenceAxes=FollowerAxes  RotationSequence=XYX  RotationSequenceAngles=[0 0 0]  RotationSequenceAnglesUnits=deg  RotationMatrix=[1 0 0;0 1 0;0 0 1]  Quaternion=[1 0 0 0]  TranslationMethod=None  TranslationCartesianOffset=[0 0 0]  TranslationCartesianOffsetUnits=m  TranslationStandardAxis=+Z  TranslationStandardOffset=0  TranslationStandardOffsetUnits=m  TranslationROffset=0  TranslationROffsetUnits=m  TranslationThetaOffset=0  TranslationThetaOffsetUnits=deg  TranslationZOffset=0  TranslationZOffsetUnits=m
  Conn1 : PMIOPort  Side=Right
  Top : PMIOPort  Port=2  Side=Left
  connections:
  Conn1/RConn1 -- Rigid Transform2/RConn1
  Revolute Joint1/RConn1 -- Rigid Transform3/LConn1
  Rigid Transform2/LConn1 -- Revolute Joint1/LConn1
  Rigid Transform3/RConn1 -- Top/RConn1
